simulink model "hyd_hyb_2_opt_cont_1_3"  (Simulink 7.7) (continued)
== system hyd_hyb_2_opt_cont_1_3/controller1/optimized controller/SOC upper 0.5/SOC under 0.6/net_loc_rod_5_6/Layer 2/LW{2,1} == (continued)
      mask Display: e = 0.06;c1 = [120 150 230]/255;c2 = ([120 150 230]+0)/383;c3 = ([120 150 230]+128)/383;patch([0 1 1 0],[0 0 1 1],c1);patch([0 1 1 1-e 1-e 0+e],[0 0 1 1-e 0+e 0+e],c2);patch([0 0 1 1-e 0+e 0+e],[0 1 1 1-e 1-e 0+e],c3);plot([0 1 1 0 0],[0 0 1 1 0]);disp('weights');
  "IW{2,1}(39,:)'" : Constant  Value=[0.051793127253268227;0.0867335350873501;-0.17123112941142279;0.28658186860972723;0.18926556937982511;-0.043935057649215618;0.13359057229240645;-0.047621375948154999;0.18793041389962434;-0.051416531487794681;-0.08856818089903655;-0.38589303828667615;-0.23467469360752585;0.32977369274502261;0.3564742972176565;0.32991702813045037;-0.31138510781671941;0.37081086613078584;0.28676153025468104;-0.30869382703943476;-0.0026450712695630205;-0.26638730282895701;0.38913376997251758;-0.14028202296315356;0.18601520036207678;-0.036540714653514224;0.14064789843506978;-0.14338190033992143;-0.34269091696785714;-0.17620658465693925;0.35632987798502669;-0.22443116239248825;0.32819725097151675;0.27936329078335781;0.081759180577006305;-0.30885710550039658;-0.45341460446711601;-0.078017544448491322;0.45253702671142459;-0.10546329101274068]
      mask Display: e = 0.06;c1 = [120 150 230]/255;c2 = ([120 150 230]+0)/383;c3 = ([120 150 230]+128)/383;patch([0 1 1 0],[0 0 1 1],c1);patch([0 1 1 1-e 1-e 0+e],[0 0 1 1-e 0+e 0+e],c2);patch([0 0 1 1-e 0+e 0+e],[0 1 1 1-e 1-e 0+e],c3);plot([0 1 1 0 0],[0 0 1 1 0]);disp('weights');
  "IW{2,1}(4,:)'" : Constant  Value=[0.26166945625251098;-0.015391374965026268;0.38843456456057973;0.20368367666233722;0.031082817938094705;-0.31984888765571673;-0.021842719752766124;0.23254936809729812;0.3733846428382005;0.17450120067696237;0.25876863857223381;-0.25021254779034552;0.30107195992017444;0.27216368076662156;0.39455419320493268;0.15055949351372644;0.18494408604779197;0.12296440911649757;0.21985512687003991;-0.20846579576787017;-0.07077424242262248;0.28168834350833466;-0.078298909476144443;-0.17164861985544724;-0.25281257372316035;0.32166865434067449;-0.18107836433143712;0.35772334437983455;0.015454886190986684;-0.3660073998703538;-0.099729487379174225;-0.34136163196315983;0.017100924014964922;0.16427923724834531;-0.38652640556843537;-0.06654457955317708;-0.36545535617732722;0.27114312057944767;-0.13280072547520147;-0.23062466165328896]
      mask Display: e = 0.06;c1 = [120 150 230]/255;c2 = ([120 150 230]+0)/383;c3 = ([120 150 230]+128)/383;patch([0 1 1 0],[0 0 1 1],c1);patch([0 1 1 1-e 1-e 0+e],[0 0 1 1-e 0+e 0+e],c2);patch([0 0 1 1-e 0+e 0+e],[0 1 1 1-e 1-e 0+e],c3);plot([0 1 1 0 0],[0 0 1 1 0]);disp('weights');
  "IW{2,1}(40,:)'" : Constant  Value=[0.14480187979545261;-0.41941714327186774;-0.35780211276912016;0.024512038372641577;0.30273435786497532;0.13878043538401386;-0.35159212406694662;-0.25398964794072676;-0.28947420623540304;0.27611795673366452;0.093365299036340638;-0.34334526523495601;0.11648410858136214;-0.1974803030154792;0.056043393075692234;-0.34591718131775501;-0.053546612480675188;0.13915966292225446;0.091872205621835665;-0.0091979654261735364;0.15727455891667225;-0.12268560560427545;-0.20861387387062075;0.28515458061013055;-0.34882815119266358;-0.27003732791152785;0.16164177881052225;0.3239620209002575;-0.23891306562469483;0.15431461217505035;0.33578879978244108;-0.26282892631903626;-0.14778316190202923;0.25256793299625435;-0.070585017727189905;-0.3006923103369778;0.24926829400645109;-0.28719001435833974;-0.12558106315396503;0.4321551170519316]
      mask Display: e = 0.06;c1 = [120 150 230]/255;c2 = ([120 150 230]+0)/383;c3 = ([120 150 230]+128)/383;patch([0 1 1 0],[0 0 1 1],c1);patch([0 1 1 1-e 1-e 0+e],[0 0 1 1-e 0+e 0+e],c2);patch([0 0 1 1-e 0+e 0+e],[0 1 1 1-e 1-e 0+e],c3);plot([0 1 1 0 0],[0 0 1 1 0]);disp('weights');
  "IW{2,1}(41,:)'" : Constant  Value=[-0.32759329641781437;0.24537346203803526;-0.0073491027998336591;0.33835466259436292;0.38067357973857574;-0.14465332964355013;0.19849777553098036;0.042958320966494948;-0.10266019858384033;0.17923280185782203;-0.31973267865946042;-0.39071311864079139;0.045577009861267302;0.39891359788374364;0.19114855963353772;0.18246140083211351;-0.061183244971679762;0.30449648647831018;-0.35902316912602578;-0.31418753242354891;-0.09119721466477837;-0.38729723428796331;-0.11000683388121742;-0.26631129317967878;0.29364103075620229;-0.27496286251298352;-0.048713715870195032;0.094191504555056982;-0.12880845984230224;0.26644173004504396;-0.32953070595739181;0.36656823302284103;-0.12231857491616857;0.066135946501131984;0.25253316837166534;-0.039780405959084469;0.16837903543925417;0.36874169374716059;0.23141980162813247;0.28029026990552897]
      mask Display: e = 0.06;c1 = [120 150 230]/255;c2 = ([120 150 230]+0)/383;c3 = ([120 150 230]+128)/383;patch([0 1 1 0],[0 0 1 1],c1);patch([0 1 1 1-e 1-e 0+e],[0 0 1 1-e 0+e 0+e],c2);patch([0 0 1 1-e 0+e 0+e],[0 1 1 1-e 1-e 0+e],c3);plot([0 1 1 0 0],[0 0 1 1 0]);disp('weights');
  "IW{2,1}(42,:)'" : Constant  Value=[-0.3087562699064354;0.01479828615981137;-0.37792834474962866;0.13677852248658373;-0.32855270204208953;-0.32325921669460389;-0.31798347467554128;0.21839109787669023;-0.31811310472283344;0.07651339820084288;0.19396724780678704;-0.38115232717214287;-0.34556257129549761;0.14007264668724193;0.15716775679286749;-0.37093964357763382;0.28224012417403421;0.14861217562703413;0.22647511515637106;-0.41652314932668055;-0.22201044225525374;-0.35206618803788309;0.085956231256808943;-0.21243863401660087;0.22773383468695901;0.22989319530872215;-0.15369937236401163;0.12308425406712353;-0.32528254046923644;-0.28972870186678595;0.054764188791439052;-0.070186872891953037;0.16638241420368713;0.34228561540854224;0.0048449502598812283;0.17060304529836359;0.21339471190757667;-0.1171274437703435;-0.35326983358716058;0.10782626260251062]
      mask Display: e = 0.06;c1 = [120 150 230]/255;c2 = ([120 150 230]+0)/383;c3 = ([120 150 230]+128)/383;patch([0 1 1 0],[0 0 1 1],c1);patch([0 1 1 1-e 1-e 0+e],[0 0 1 1-e 0+e 0+e],c2);patch([0 0 1 1-e 0+e 0+e],[0 1 1 1-e 1-e 0+e],c3);plot([0 1 1 0 0],[0 0 1 1 0]);disp('weights');
  "IW{2,1}(43,:)'" : Constant  Value=[-0.27671403381418425;0.39497644529486614;0.19884039529498637;-0.024207540201849165;0.3368193130313426;-0.047785363384455118;-0.053348280601332544;0.19191831154073752;-0.28904321521883608;-0.18790579654020279;-0.29625544077472371;-0.24353234951426797;0.099944071485222427;-0.23679764618180843;0.10147430907766201;-0.22027818586511319;0.13584341912346798;-0.25231661471105238;0.25091050704522933;-0.010072938457797123;0.3291327918487657;-0.23398288711799142;-0.36794194806688096;0.010433704228146969;0.1001893720398319;-0.025734088154408617;-0.013144305920785056;-0.13425225101814162;0.29273211599546078;0.35289104932516746;0.32854602239118469;0.3692239932887314;0.3393458848165119;0.10909001070815789;-0.41059103135578612;-0.23851373264167422;0.25116970359433449;-0.21895921531569695;-0.30035182657892689;0.28160258355361861]
      mask Display: e = 0.06;c1 = [120 150 230]/255;c2 = ([120 150 230]+0)/383;c3 = ([120 150 230]+128)/383;patch([0 1 1 0],[0 0 1 1],c1);patch([0 1 1 1-e 1-e 0+e],[0 0 1 1-e 0+e 0+e],c2);patch([0 0 1 1-e 0+e 0+e],[0 1 1 1-e 1-e 0+e],c3);plot([0 1 1 0 0],[0 0 1 1 0]);disp('weights');
  "IW{2,1}(44,:)'" : Constant  Value=[0.065227653002081581;0.37567576744042125;0.20103320961503496;-0.11232882676002968;0.22422593395505538;-0.25013990568364203;0.3976615741642005;0.18900272741947399;-0.21543208604527805;-0.28026511530584153;0.23215890846487516;0.1381062311135694;0.35403485176579924;0.028443255421127717;0.20080551666925714;0.38630286398688396;-0.11558036534184107;0.39335657481233294;0.096464815943382243;-0.070012555922095834;0.18874144124451095;0.23482754072367579;-0.13860133741739722;0.38263286223379006;0.094141169405718206;0.34729770353663736;0.33682673033033583;-0.29747893504632267;-0.34466577024137113;0.010764802663778055;0.17222163154614753;0.20601103947969931;0.14521477526533227;0.11364023619482251;-0.13893097635497781;-0.25249547970694969;0.38702438248298654;0.28124833771607732;0.2124379891606395;-0.18291814755523148]
      mask Display: e = 0.06;c1 = [120 150 230]/255;c2 = ([120 150 230]+0)/383;c3 = ([120 150 230]+128)/383;patch([0 1 1 0],[0 0 1 1],c1);patch([0 1 1 1-e 1-e 0+e],[0 0 1 1-e 0+e 0+e],c2);patch([0 0 1 1-e 0+e 0+e],[0 1 1 1-e 1-e 0+e],c3);plot([0 1 1 0 0],[0 0 1 1 0]);disp('weights');
  "IW{2,1}(45,:)'" : Constant  Value=[-0.081464031813616902;0.238240758316832;0.0042004367062321701;0.18683306169738675;0.22263586522550124;0.29398394728067018;0.28752148452180298;0.26764571612217769;-0.23729322869172653;-0.41556721490699555;0.32126348780488395;0.27007773946099162;-0.023783747835480228;0.29204046065570788;0.26844587062248099;0.27644499197004113;-0.13109666364143963;-0.23911534642184273;-0.39783838378068603;-0.2170966916107597;0.13375792636947173;0.16375513787908644;-0.014400969191436644;0.39863553541260921;0.43155932817639975;-0.26665508454510833;-0.39251517147691362;0.14086978576014386;-0.28151832150872846;0.17177495014893215;-0.032674953675965658;-0.15710461227402442;-0.11630343444076759;-0.032597086898104741;0.26770855307164854;0.1282672531941387;-0.17989830173013766;0.49053183100815639;0.12475772560683679;-0.26983163749503158]
      mask Display: e = 0.06;c1 = [120 150 230]/255;c2 = ([120 150 230]+0)/383;c3 = ([120 150 230]+128)/383;patch([0 1 1 0],[0 0 1 1],c1);patch([0 1 1 1-e 1-e 0+e],[0 0 1 1-e 0+e 0+e],c2);patch([0 0 1 1-e 0+e 0+e],[0 1 1 1-e 1-e 0+e],c3);plot([0 1 1 0 0],[0 0 1 1 0]);disp('weights');
  "IW{2,1}(46,:)'" : Constant  Value=[-0.3131015924298437;0.28708141016942584;-0.017940407307627852;0.12302802936240229;0.015801980627966072;-0.25749936710593385;-0.0017638941688190601;-0.048466674773344154;0.12367087849598908;-0.28411625991077677;0.41300423953876486;0.19804327765225599;-0.0060259105922243891;0.11288894515416228;-0.27024714482357415;0.40065325810964381;-0.18774569556601342;-0.34864070171139888;-0.11793485572002647;-0.34886355389266188;0.041940135584584037;-0.2986546419404244;-0.032786726596024093;0.33284337085533011;-0.43564516977184942;0.19356169082536467;-0.053462716786198641;0.2689344351354116;0.25158389968106465;-0.12519570265187002;0.24248964573726503;0.34709656011161522;-0.36725357003256975;-0.22030911861624797;0.19865683130496931;0.033097178620337037;-0.38746058008191347;-0.03520749260065742;0.28124866971127938;-0.12293306442183935]
      mask Display: e = 0.06;c1 = [120 150 230]/255;c2 = ([120 150 230]+0)/383;c3 = ([120 150 230]+128)/383;patch([0 1 1 0],[0 0 1 1],c1);patch([0 1 1 1-e 1-e 0+e],[0 0 1 1-e 0+e 0+e],c2);patch([0 0 1 1-e 0+e 0+e],[0 1 1 1-e 1-e 0+e],c3);plot([0 1 1 0 0],[0 0 1 1 0]);disp('weights');
  "IW{2,1}(47,:)'" : Constant  Value=[-0.28084672853866371;0.029521095852503572;0.098594644171722048;0.34540163619638953;-0.22640949693072179;0.13310969295224881;0.11174150600891639;-0.2479231339953944;-0.32978391677739266;0.24041616470301058;-0.20251081540029015;0.038090352038501168;-0.25684787491728123;0.24060913613006474;0.40993175496267253;0.45032100795897795;-0.12640075179132948;-0.083741955962267134;-0.13863430319698547;-0.34441848480356158;-0.41165610188065399;-0.26833870881883193;0.19719285230884151;-0.21292078103941722;0.27736549258419557;-0.22561486601889616;-0.35397775628179223;0.003205401375338882;-0.30596818862743269;0.067211218106433213;-0.27497640230046944;0.0039137518864756264;-0.24483615244421036;0.47319103764171394;-0.0068306541671852329;0.21029192332308363;-0.37492174428040442;-0.021206360167852861;0.26184641848369461;-0.12139101255231555]
      mask Display: e = 0.06;c1 = [120 150 230]/255;c2 = ([120 150 230]+0)/383;c3 = ([120 150 230]+128)/383;patch([0 1 1 0],[0 0 1 1],c1);patch([0 1 1 1-e 1-e 0+e],[0 0 1 1-e 0+e 0+e],c2);patch([0 0 1 1-e 0+e 0+e],[0 1 1 1-e 1-e 0+e],c3);plot([0 1 1 0 0],[0 0 1 1 0]);disp('weights');
  "IW{2,1}(48,:)'" : Constant  Value=[-0.2643355616865401;0.34425478114156233;-0.16244865797822977;0.178870552639351;0.15919268671323944;-0.12878378785634878;-0.011951761272175;0.34005816541828277;-0.31406200901536557;-0.36470646467168305;0.37879476345470459;0.34297802880043166;0.14710579412967234;-0.34580216476294418;-0.20861202093781545;0.11893405614546605;0.04261443931693143;-0.13459346405537501;0.062439473613574228;0.22576278634134267;-0.072723684122369819;0.024705465938471109;-0.22770058818618302;0.25354789746539008;0.13772958556774628;0.3892125159691745;0.31032738665266585;-0.073210635317150538;-0.28253162154338313;0.34918467511879187;-0.16869301916580767;-0.28798050605717312;0.37158583474338197;-0.19078079185200386;-0.34518181474522325;-0.09500862056339987;-0.084125022847577821;0.31054245830317218;-0.24142393580183538;-0.24517505407646173]
      mask Display: e = 0.06;c1 = [120 150 230]/255;c2 = ([120 150 230]+0)/383;c3 = ([120 150 230]+128)/383;patch([0 1 1 0],[0 0 1 1],c1);patch([0 1 1 1-e 1-e 0+e],[0 0 1 1-e 0+e 0+e],c2);patch([0 0 1 1-e 0+e 0+e],[0 1 1 1-e 1-e 0+e],c3);plot([0 1 1 0 0],[0 0 1 1 0]);disp('weights');
  "IW{2,1}(49,:)'" : Constant  Value=[-0.10369218368525379;0.16049968045997356;0.1370999202076566;-0.14068193172798396;-0.32437800383064735;0.12048793745400112;-0.13797528738723377;0.4102663384015976;-0.24631564072981954;0.30390743779640328;0.39272975578494773;0.19969541433908775;-0.093264804300024012;-0.23443820833569404;0.055413302705005239;-0.17859731531426826;0.04653566335901986;-0.14671876821235266;-0.24221939829727179;0.14910638093631309;-0.33183066118989824;0.082210028961534246;0.27882495191162654;-0.21029055917758854;0.34852904568376464;-0.20302484038710267;0.35764846378407955;-0.23996418745827103;0.20732496543276474;0.21107884939281563;0.20400430449168336;-0.080346502359988109;-0.054689476995769093;-0.23242548780761571;0.26758456994354907;-0.42923725932552476;-0.40662572287826104;0.273036853482237;-0.2722266549908321;0.36658922899384533]
      mask Display: e = 0.06;c1 = [120 150 230]/255;c2 = ([120 150 230]+0)/383;c3 = ([120 150 230]+128)/383;patch([0 1 1 0],[0 0 1 1],c1);patch([0 1 1 1-e 1-e 0+e],[0 0 1 1-e 0+e 0+e],c2);patch([0 0 1 1-e 0+e 0+e],[0 1 1 1-e 1-e 0+e],c3);plot([0 1 1 0 0],[0 0 1 1 0]);disp('weights');
  "IW{2,1}(5,:)'" : Constant  Value=[0.16055742864868752;0.36647416856062442;0.26446171161236554;0.045070009581576156;0.15199860658008635;0.44335938921561779;0.26738569053125205;-0.12507279109476832;0.33777030918327716;0.24408779449840684;-0.32557199370441714;-0.028932838115301413;0.34436046249352126;-0.12927259289299986;0.35628383106581446;-0.024878291249822954;-0.14238023709580463;0.083958595830212493;-0.089800245230982387;0.22469375917611878;-0.17502194675290383;-0.35678667859132274;0.40091962586710916;-0.098554991294222655;-0.31773333728495701;0.2562016293755075;-0.22645681774596871;-0.30415772206997094;-0.099050001842588581;-0.094775146738007424;-0.1757442165413495;0.24821333940970255;-0.1958521430006073;0.18327496318528899;0.12174265153894498;0.23502474015238597;0.19156809126273147;0.29201913670839935;-0.41021772384697747;0.17114587739684597]
      mask Display: e = 0.06;c1 = [120 150 230]/255;c2 = ([120 150 230]+0)/383;c3 = ([120 150 230]+128)/383;patch([0 1 1 0],[0 0 1 1],c1);patch([0 1 1 1-e 1-e 0+e],[0 0 1 1-e 0+e 0+e],c2);patch([0 0 1 1-e 0+e 0+e],[0 1 1 1-e 1-e 0+e],c3);plot([0 1 1 0 0],[0 0 1 1 0]);disp('weights');
  "IW{2,1}(50,:)'" : Constant  Value=[-0.15281389713634788;0.19062419672170808;-0.34764931787619924;0.27514251865922756;-0.19328601621349023;-0.040597910444059422;-0.40753293783037459;0.21786977827475859;0.20925930737481604;-0.28169193279566002;0.17219248733535616;-0.16653941575701306;0.071061364288123319;-0.38187207662334305;0.05341160547795501;-0.030269865651755878;0.37274176929633573;-0.19809485756408182;0.10354903983592244;-0.18677378857905305;-0.28076214066856958;-0.42442416308118375;0.093905929614936642;-0.01647997833014337;0.19725398594495674;0.28573663385018105;-0.32239719892111185;-0.23563869743960991;-0.27987425149980466;-0.31546418426822903;-0.092061435590861981;0.31952885470934816;0.26531139372623153;0.37589704024124276;0.25525597751551365;-0.33819397117131333;0.20735648181858146;0.045550050317796797;-0.22467748206766908;-0.024869856971619048]
      mask Display: e = 0.06;c1 = [120 150 230]/255;c2 = ([120 150 230]+0)/383;c3 = ([120 150 230]+128)/383;patch([0 1 1 0],[0 0 1 1],c1);patch([0 1 1 1-e 1-e 0+e],[0 0 1 1-e 0+e 0+e],c2);patch([0 0 1 1-e 0+e 0+e],[0 1 1 1-e 1-e 0+e],c3);plot([0 1 1 0 0],[0 0 1 1 0]);disp('weights');
  "IW{2,1}(51,:)'" : Constant  Value=[-0.044834129482377708;-0.28596644997621035;-0.36638378579618314;-0.0091342195695461384;-0.047353762506909883;0.20328889975965753;0.3196062127309488;0.12863310425025226;0.22268708878896676;-0.10533287251400732;0.14453075855950201;-0.013761665837647791;-0.2423664827033524;-0.34345544612576595;0.15259539876913683;-0.37720175340548079;0.15663417235559088;-0.39611062678353115;-0.27866218619558741;-0.060955366354394587;-0.34109232890590802;-0.17640795920486993;0.068692453924288271;-0.41138918674168018;-0.37631516864547526;0.26772645372628145;-0.25863977450137327;-0.2194814658292227;0.38857383268480122;0.2810395270458787;-0.099899804068713974;-0.17557502697715141;-0.03784197548576889;-0.14169457715621939;0.22382566406576296;-0.37536334052825243;-0.11570770939274132;0.21199516775718522;-0.072539333431306974;0.41058533232639743]
      mask Display: e = 0.06;c1 = [120 150 230]/255;c2 = ([120 150 230]+0)/383;c3 = ([120 150 230]+128)/383;patch([0 1 1 0],[0 0 1 1],c1);patch([0 1 1 1-e 1-e 0+e],[0 0 1 1-e 0+e 0+e],c2);patch([0 0 1 1-e 0+e 0+e],[0 1 1 1-e 1-e 0+e],c3);plot([0 1 1 0 0],[0 0 1 1 0]);disp('weights');
  "IW{2,1}(52,:)'" : Constant  Value=[0.29610226928355099;-0.016551889878568649;-0.18573678716326819;0.29365682735367643;-0.31675995480847857;-0.015600600275043532;-0.060557818124216879;0.23340598471150198;-0.055530868688486605;-0.45873372283063224;0.24379819836883376;-0.20383677474585624;0.01605275450075808;-0.093957924037893506;0.20120718185948991;0.034062683585666075;-0.22579940162047293;-0.42600810178110154;-0.37794399384082322;-0.12234782496674226;0.28978146267238364;-0.17660860112317084;-0.042872108592497807;-0.094295931752574516;-0.079834831585061578;0.40282897987164401;0.16622519001995781;0.24193390458753442;-0.24712922873773424;0.36189046547473175;0.34920423861945071;0.13106308355432403;0.22539319235041635;0.31902293455093284;0.28367553675032048;0.22145515159650239;0.033533241739290634;0.30736674793031216;0.31973069897255885;0.3995242330755947]
      mask Display: e = 0.06;c1 = [120 150 230]/255;c2 = ([120 150 230]+0)/383;c3 = ([120 150 230]+128)/383;patch([0 1 1 0],[0 0 1 1],c1);patch([0 1 1 1-e 1-e 0+e],[0 0 1 1-e 0+e 0+e],c2);patch([0 0 1 1-e 0+e 0+e],[0 1 1 1-e 1-e 0+e],c3);plot([0 1 1 0 0],[0 0 1 1 0]);disp('weights');
  "IW{2,1}(53,:)'" : Constant  Value=[-0.11453628898764484;0.40004253573854115;-0.10329560945856374;-0.06568419998566831;-0.14006989641667045;0.11414706558182737;0.36250888389079289;-0.026271731333233746;0.28565770583722361;0.29586454091840519;0.11640137077308103;-0.36668867447573794;-0.23936084675278718;-0.41688021547575238;-0.14492637012579851;-0.21536351483916288;0.44445485025045478;-0.19143427328143597;0.1295561139733967;0.33915378242214911;-0.25260513471644541;0.22363113778798399;-0.16875298893285698;0.29108506296618714;0.34560699685480839;0.27092584503998424;-0.23365303962946501;0.28010670409068811;-0.19228248819718435;-0.010467387166723293;0.224998200538372;0.086826364033216474;-0.26960210679307922;-0.24600525766496167;-0.32694370689473184;0.27708909509041207;0.39490336064886467;-0.14298158353938634;0.12440347582115165;0.36602672096102334]
      mask Display: e = 0.06;c1 = [120 150 230]/255;c2 = ([120 150 230]+0)/383;c3 = ([120 150 230]+128)/383;patch([0 1 1 0],[0 0 1 1],c1);patch([0 1 1 1-e 1-e 0+e],[0 0 1 1-e 0+e 0+e],c2);patch([0 0 1 1-e 0+e 0+e],[0 1 1 1-e 1-e 0+e],c3);plot([0 1 1 0 0],[0 0 1 1 0]);disp('weights');
  "IW{2,1}(54,:)'" : Constant  Value=[-0.33217508339050139;-0.13900554547754446;-0.37607459436904628;0.29417499618771731;-0.011008838896999123;0.021037088807147521;0.11494685360538809;0.0095844321318628023;-0.35207481288704001;-0.47161335401532828;0.42605554140589624;0.44103583338038244;-0.15468903316988117;-0.20114737199132252;-0.026692682681089968;0.017953861455726763;0.090842894224184748;0.2037560645287628;0.38461167827661502;-0.104633195591192;-0.17567422227113472;0.059787155927483716;-0.3660568853190852;0.1015274881005553;-0.31665601473051253;-0.30713376022254718;0.091826944381655437;-0.30169329509841297;0.4327225469202331;0.29908695812390462;0.015494907426369855;0.18061055372520179;0.1042586247075392;-0.074092415641255238;0.28060243793417827;0.0071399279844863005;-0.068007582463831956;0.34252453656393284;-0.12492786083026403;0.13221581067883578]
      mask Display: e = 0.06;c1 = [120 150 230]/255;c2 = ([120 150 230]+0)/383;c3 = ([120 150 230]+128)/383;patch([0 1 1 0],[0 0 1 1],c1);patch([0 1 1 1-e 1-e 0+e],[0 0 1 1-e 0+e 0+e],c2);patch([0 0 1 1-e 0+e 0+e],[0 1 1 1-e 1-e 0+e],c3);plot([0 1 1 0 0],[0 0 1 1 0]);disp('weights');
  "IW{2,1}(55,:)'" : Constant  Value=[0.13206316868607926;-0.13095557376853312;-0.25427119284544281;0.16585857909784729;-0.19413563821325741;-0.060397247828158022;-0.20851166201635471;-0.12026430379917294;0.39355926784507878;0.23558551915172071;-0.31280172049654992;0.18047811280237352;0.38885718028549437;0.33192572102844931;0.39672857357165592;0.28451986184763772;0.36451888965701529;0.17893038241470585;0.38102315780041568;0.1737787185394799;-0.039017142135691234;0.011242315456324599;0.066983506148263358;0.17068670579249215;-0.32249070558685455;0.37708381420735976;-0.32540021659205287;0.33333967816623239;-0.03899088566454996;-0.23683832177967296;-0.20803120344492382;-0.12788047104548614;-0.34422044322861517;-0.049597990506248045;0.056994532122713208;-0.13206724470605;-0.078789912300635312;0.32798456273540216;0.18180876531643475;0.32959084862643184]
      mask Display: e = 0.06;c1 = [120 150 230]/255;c2 = ([120 150 230]+0)/383;c3 = ([120 150 230]+128)/383;patch([0 1 1 0],[0 0 1 1],c1);patch([0 1 1 1-e 1-e 0+e],[0 0 1 1-e 0+e 0+e],c2);patch([0 0 1 1-e 0+e 0+e],[0 1 1 1-e 1-e 0+e],c3);plot([0 1 1 0 0],[0 0 1 1 0]);disp('weights');
  "IW{2,1}(56,:)'" : Constant  Value=[0.43449351604480108;0.38128023742120931;-0.23715988580581598;0.0034285443848455152;-0.3051004183029547;-0.010840640382541532;-0.43463272534441871;0.43360379352972267;-0.47401339356254085;-0.22717740700111155;-0.32880141219397341;-0.46746441472073702;-0.094154298119462035;0.1080330781544028;-0.065269896142724826;-0.044342734538179288;-0.29463254778187753;0.30431942490484737;0.17744253731969264;0.19342950120999733;0.28524128235621254;-0.07135889342569135;0.26075386204060325;0.1914434599099781;-0.005588241421249235;-0.27680645487068939;-0.14977796748975727;-0.16475105417707767;-0.21408000379735725;-0.07438968395935186;-0.13674629476099984;-0.036473030969233954;0.01341551002289708;0.048761102260615197;-0.12396321914253287;0.2447598942929545;-0.44486382694406007;0.016716694621365089;-0.19559748776131355;0.22025685868904515]
      mask Display: e = 0.06;c1 = [120 150 230]/255;c2 = ([120 150 230]+0)/383;c3 = ([120 150 230]+128)/383;patch([0 1 1 0],[0 0 1 1],c1);patch([0 1 1 1-e 1-e 0+e],[0 0 1 1-e 0+e 0+e],c2);patch([0 0 1 1-e 0+e 0+e],[0 1 1 1-e 1-e 0+e],c3);plot([0 1 1 0 0],[0 0 1 1 0]);disp('weights');
  "IW{2,1}(57,:)'" : Constant  Value=[-0.0083745728553244608;0.29476685390391549;0.33262077914476029;0.19653322562095363;0.11454207185404543;-0.34322863929539421;-0.35467575257568884;-0.03054196394628924;-0.31679789362858796;-0.39207677450342338;0.33695879919731436;-0.04593405346293996;0.15488457175891668;-0.37684760863890887;0.1746759653597047;-0.26313310839845239;-0.059354532981901319;0.14331580228799276;0.36797040752388466;-0.34510857449381083;-0.14815903465579042;0.033453469056473471;-0.045638582246678251;0.058509696521560982;-0.05021381888045038;-0.0067222434703053295;-0.30128993564822959;-0.32936309309338674;-0.38911690857055375;0.039634825934388114;0.32105537833767361;0.048933868343075462;0.28927717855369434;0.0099448095300426077;0.1102932069666879;-0.32637681778583011;0.35757459269816311;-0.25620996846653871;-0.32405846714956377;-0.085668009180640298]
      mask Display: e = 0.06;c1 = [120 150 230]/255;c2 = ([120 150 230]+0)/383;c3 = ([120 150 230]+128)/383;patch([0 1 1 0],[0 0 1 1],c1);patch([0 1 1 1-e 1-e 0+e],[0 0 1 1-e 0+e 0+e],c2);patch([0 0 1 1-e 0+e 0+e],[0 1 1 1-e 1-e 0+e],c3);plot([0 1 1 0 0],[0 0 1 1 0]);disp('weights');
  "IW{2,1}(58,:)'" : Constant  Value=[-0.29149125785979185;0.08070714033442565;0.18812190120955219;-0.32638238696815947;0.38728763150981282;-0.37981586507889092;0.12978590289611999;0.039839413213652135;0.12020705201532997;-0.21499443701212853;-0.21568302375121201;0.2388640226988322;-0.30151231575673948;0.15814161811198985;-0.21389779803994363;-0.32632225430957623;0.34302701855415901;0.34504545467679826;0.044895005422193074;-0.12456127431860828;0.22485787027609644;-0.30590200526019479;0.31533177007694524;0.25921948097591446;0.26133261740208641;-0.31973508913637372;-0.25466428104923977;-0.17674476733364849;0.20477978987733522;-0.014160069884654149;0.27715588362438609;-0.11520482262955282;-0.39679291730427879;-0.3401412558818635;-0.10844563083706397;-0.10863543881238494;0.22228821929161124;0.084587878752809084;-0.16412792753187838;-0.28639142307178106]
      mask Display: e = 0.06;c1 = [120 150 230]/255;c2 = ([120 150 230]+0)/383;c3 = ([120 150 230]+128)/383;patch([0 1 1 0],[0 0 1 1],c1);patch([0 1 1 1-e 1-e 0+e],[0 0 1 1-e 0+e 0+e],c2);patch([0 0 1 1-e 0+e 0+e],[0 1 1 1-e 1-e 0+e],c3);plot([0 1 1 0 0],[0 0 1 1 0]);disp('weights');
  "IW{2,1}(59,:)'" : Constant  Value=[0.25636457724538558;-0.04064749452283091;-0.0032783363947652745;-0.32820881605643004;0.29550135018036755;0.082109254120601119;0.25634018646348777;-0.45102079566371212;0.33414575457472556;0.13266312345187581;0.34490489495719295;0.36644779337044725;0.013750974679295831;-0.33828290859329607;-0.3905598154070718;-0.07007745382513153;0.085127922496425729;0.089359162004555112;0.1662071850282994;0.19170161137339073;0.012818803846722729;0.006431316033630465;-0.16379869918044762;-0.065888801552330609;-0.40870016063158482;-0.32373690208955758;-0.39482534237541811;-0.16696615293893427;-0.23727199757621775;0.33879704775218639;-0.35751485051084786;0.0014770915992821005;0.12218129385359579;0.10427221192686932;-0.37009207022807816;0.29829437429388539;-0.28677958990240626;-0.10146392730908625;-0.17317631377829892;-0.019812352015267407]
      mask Display: e = 0.06;c1 = [120 150 230]/255;c2 = ([120 150 230]+0)/383;c3 = ([120 150 230]+128)/383;patch([0 1 1 0],[0 0 1 1],c1);patch([0 1 1 1-e 1-e 0+e],[0 0 1 1-e 0+e 0+e],c2);patch([0 0 1 1-e 0+e 0+e],[0 1 1 1-e 1-e 0+e],c3);plot([0 1 1 0 0],[0 0 1 1 0]);disp('weights');
  "IW{2,1}(6,:)'" : Constant  Value=[-0.30955969531719529;-0.10986787442712617;-0.26712501428413177;0.36541133761382433;-0.37076743912507021;-0.30847618385933828;-0.018189648381940888;-0.077448410772225904;0.34656098503243726;0.25381301284008684;0.15956546972031357;0.32999981244395071;-0.36498872624056944;0.29096500009313897;0.17905588115582044;0.30778659813020443;0.067636679121266793;0.029546116665996609;0.1557311981750131;-0.31540397844205859;-0.10743144340970409;-0.38539202689069196;0.036767637542075002;0.29894030770100166;0.06008732746203397;-0.34084731684653208;0.044816122341888462;-0.28290816056823664;-0.23617670531916327;0.40696764036386268;-0.22484896947516056;0.16558883966127932;0.14073760442608821;0.19511862745258216;0.18901276988659418;0.060092308497508184;-0.25547094347016291;0.27116250314831297;-0.13806582680663976;0.036929388514358535]
      mask Display: e = 0.06;c1 = [120 150 230]/255;c2 = ([120 150 230]+0)/383;c3 = ([120 150 230]+128)/383;patch([0 1 1 0],[0 0 1 1],c1);patch([0 1 1 1-e 1-e 0+e],[0 0 1 1-e 0+e 0+e],c2);patch([0 0 1 1-e 0+e 0+e],[0 1 1 1-e 1-e 0+e],c3);plot([0 1 1 0 0],[0 0 1 1 0]);disp('weights');
  "IW{2,1}(60,:)'" : Constant  Value=[-0.10219728922827656;-0.26727183264254656;0.063116849797940633;0.13477773911427879;0.060254065703592923;0.2442530329419044;-0.22422261113437139;-0.17624133107662213;0.37424127439873606;-0.21049410308949676;0.29892696477958852;0.32308017098279673;0.21704915283409237;0.10125818751998972;-0.40117401015295284;-0.21530980711315945;0.33212505105061596;0.27003743548852072;-0.014563905297295454;-0.043428819802400528;0.31941419862810361;-0.30479846644638425;0.061452001461875475;0.31929334683281191;-0.33356094787898188;-0.27740523037167536;-0.14022605703943641;0.16827616224608777;0.32578062066471181;0.026608232911129428;-0.21994363685375756;0.39159423618004324;-0.37979117957367875;0.098682612362646124;-0.37466272499700515;0.37209002458882035;0.17020068457114118;0.21041230683435286;0.29510908416786436;-0.1438470021765825]
      mask Display: e = 0.06;c1 = [120 150 230]/255;c2 = ([120 150 230]+0)/383;c3 = ([120 150 230]+128)/383;patch([0 1 1 0],[0 0 1 1],c1);patch([0 1 1 1-e 1-e 0+e],[0 0 1 1-e 0+e 0+e],c2);patch([0 0 1 1-e 0+e 0+e],[0 1 1 1-e 1-e 0+e],c3);plot([0 1 1 0 0],[0 0 1 1 0]);disp('weights');
  "IW{2,1}(7,:)'" : Constant  Value=[0.13354653953659223;0.3687102365019958;0.28634862125021898;0.37684569823125363;0.22401738867012258;-0.022765324816638257;-0.37674725261436232;-0.25929014529613892;0.20582038860585583;0.15751709049831142;-0.25345829414238114;-0.26190484864752317;0.23199371932912036;0.19223385058274312;-0.084534901096333825;-0.12675685387213104;-0.16009602570550296;-0.32112629772416001;-0.13202760819357082;-0.19691365094924096;-0.37979246584836551;0.40510270357279216;0.30860116703203588;-0.27162714509813368;0.19657628326827717;-0.15909309143245701;-0.32609194315055912;-0.048279870580227029;0.086797250536747167;0.3447393758161828;-0.045600036418953535;0.14544476088068897;0.10774615448023141;0.32513421600812498;0.23353759451311992;0.37788876770981883;0.13299211865478869;0.20631139028328302;0.12903718678604056;-0.27507249345165086]
      mask Display: e = 0.06;c1 = [120 150 230]/255;c2 = ([120 150 230]+0)/383;c3 = ([120 150 230]+128)/383;patch([0 1 1 0],[0 0 1 1],c1);patch([0 1 1 1-e 1-e 0+e],[0 0 1 1-e 0+e 0+e],c2);patch([0 0 1 1-e 0+e 0+e],[0 1 1 1-e 1-e 0+e],c3);plot([0 1 1 0 0],[0 0 1 1 0]);disp('weights');
  "IW{2,1}(8,:)'" : Constant  Value=[0.28746083212739132;0.44766539390378596;0.41629772437026624;0.18453488579142421;0.28883808518962917;0.17297342704773938;0.041647382702848547;-0.16984459105530275;0.21308075830960901;0.2665141047141249;-0.33214626248781121;0.23874313706826863;0.13676062172713566;-0.33947436100650707;-0.1102932718565028;0.0032730066386971808;-0.20144134038583789;0.014449116872080416;0.23762957562646495;-0.35772287898530952;-0.37818368887515041;0.15800214658819686;-0.12626154682898322;0.17291279448175234;-0.32147595246229332;0.23545411246497983;0.065031361903296628;-0.1286026352211605;0.15838575220360729;0.22152273860313679;0.3586901246398071;-0.17680198739051894;-0.18767348272978962;0.024173063048223736;-0.02437453649748941;0.21220206517456908;0.24549673404579961;0.29548756457549885;-0.27836934225209808;-0.41236633110503151]
      mask Display: e = 0.06;c1 = [120 150 230]/255;c2 = ([120 150 230]+0)/383;c3 = ([120 150 230]+128)/383;patch([0 1 1 0],[0 0 1 1],c1);patch([0 1 1 1-e 1-e 0+e],[0 0 1 1-e 0+e 0+e],c2);patch([0 0 1 1-e 0+e 0+e],[0 1 1 1-e 1-e 0+e],c3);plot([0 1 1 0 0],[0 0 1 1 0]);disp('weights');
  "IW{2,1}(9,:)'" : Constant  Value=[0.056421987938368445;0.43051209121193801;-0.3177636045668269;0.015195481542260436;0.002009567801928363;-0.12643216616039726;0.11040480047567242;-0.29921226080484603;0.13166219139945651;-0.42419942446073738;-0.26276328874254928;0.01241966952745441;0.038295682332895167;-0.27949392557675939;-0.19140643956849898;-0.2423638287239458;-0.4384301356288024;0.075895123224735059;-0.25948247928180085;-0.12059537446297611;-0.39135991625943956;0.24631872462321625;-0.094520233292546371;0.25762259679894017;0.19321668787834798;0.32673708796781814;-0.3017716102427998;0.058908786729054592;-0.39175769668372978;0.36788628681445579;0.068188774178362679;-0.08094218960639224;0.13526100636190302;-0.23359756637694429;-0.22104449204308332;0.38049963094761075;-0.26220535878296353;-0.074783723679575864;-0.30623974935948578;-0.29893377007521543]
      mask Display: e = 0.06;c1 = [120 150 230]/255;c2 = ([120 150 230]+0)/383;c3 = ([120 150 230]+128)/383;patch([0 1 1 0],[0 0 1 1],c1);patch([0 1 1 1-e 1-e 0+e],[0 0 1 1-e 0+e 0+e],c2);patch([0 0 1 1-e 0+e 0+e],[0 1 1 1-e 1-e 0+e],c3);plot([0 1 1 0 0],[0 0 1 1 0]);disp('weights');
  Mux : Mux  Inputs=60
      mask Display: e = 0.06;c1 = [120 150 230]/255;c2 = ([120 150 230]+0)/383;c3 = ([120 150 230]+128)/383;patch([0 1 1 0],[0 0 1 1],c1);patch([0 1 1 1-e 1-e 0+e],[0 0 1 1-e 0+e 0+e],c2);patch([0 0 1 1-e 0+e 0+e],[0 1 1 1-e 1-e 0+e],c3);plot([0 1 1 0 0],[0 0 1 1 0]);disp('Mux');
  dotprod{1..60} (60 alike) : neural/Weight Functions/dotprod  SystemSampleTime=-1
  "lz{2,1}" : Outport
      mask Display: e = 0.06;c1 = [120 230 180]/255;c2 = ([120 230 180]+0)/(256+128);c3 = ([120 230 180]+128)/(256+128);patch([0 1 1 0],[0 0 1 1],c1);patch([0 1 1 1-e 1-e 0+e],[0 0 1 1-e 0+e 0+e],c2);patch([0 0 1 1-e 0+e 0+e],[0 1 1 1-e 1-e 0+e],c3);plot([0 1 1 0 0],[0 0 1 1 0]);
  wires:
  "IW{2,1}(1,:)'"/1 -> dotprod1/1
  "ad{2,1}"/1 -> dotprod1/2, dotprod2/2, dotprod3/2, dotprod4/2, dotprod5/2, dotprod6/2, dotprod7/2, dotprod8/2, dotprod9/2, dotprod10/2, dotprod11/2, dotprod12/2, dotprod13/2, dotprod14/2, dotprod15/2, dotprod16/2, dotprod17/2, dotprod18/2, dotprod19/2, dotprod20/2, dotprod21/2, dotprod22/2, dotprod23/2, dotprod24/2, dotprod25/2, dotprod26/2, dotprod27/2, dotprod28/2, dotprod29/2, dotprod30/2, dotprod31/2, dotprod32/2, dotprod33/2, dotprod34/2, dotprod35/2, dotprod36/2, dotprod37/2, dotprod38/2, dotprod39/2, dotprod40/2, dotprod41/2, dotprod42/2, dotprod43/2, dotprod44/2, dotprod45/2, dotprod46/2, dotprod47/2, dotprod48/2, dotprod49/2, dotprod50/2, dotprod51/2, dotprod52/2, dotprod53/2, dotprod54/2, dotprod55/2, dotprod56/2, dotprod57/2, dotprod58/2, dotprod59/2, dotprod60/2
  dotprod1/1 -> Mux/1
  "IW{2,1}(2,:)'"/1 -> dotprod2/1
  dotprod2/1 -> Mux/2
  "IW{2,1}(3,:)'"/1 -> dotprod3/1
  dotprod3/1 -> Mux/3
  "IW{2,1}(4,:)'"/1 -> dotprod4/1
  dotprod4/1 -> Mux/4
  "IW{2,1}(5,:)'"/1 -> dotprod5/1
  dotprod5/1 -> Mux/5
  "IW{2,1}(6,:)'"/1 -> dotprod6/1
  dotprod6/1 -> Mux/6
  "IW{2,1}(7,:)'"/1 -> dotprod7/1
  dotprod7/1 -> Mux/7
  "IW{2,1}(8,:)'"/1 -> dotprod8/1
  dotprod8/1 -> Mux/8
  "IW{2,1}(9,:)'"/1 -> dotprod9/1
  dotprod9/1 -> Mux/9
  "IW{2,1}(10,:)'"/1 -> dotprod10/1
  dotprod10/1 -> Mux/10
  "IW{2,1}(11,:)'"/1 -> dotprod11/1
  dotprod11/1 -> Mux/11
  "IW{2,1}(12,:)'"/1 -> dotprod12/1
  dotprod12/1 -> Mux/12
  "IW{2,1}(13,:)'"/1 -> dotprod13/1
  dotprod13/1 -> Mux/13
  "IW{2,1}(14,:)'"/1 -> dotprod14/1
  dotprod14/1 -> Mux/14
  "IW{2,1}(15,:)'"/1 -> dotprod15/1
  dotprod15/1 -> Mux/15
  "IW{2,1}(16,:)'"/1 -> dotprod16/1
  dotprod16/1 -> Mux/16
  "IW{2,1}(17,:)'"/1 -> dotprod17/1
  dotprod17/1 -> Mux/17
  "IW{2,1}(18,:)'"/1 -> dotprod18/1
  dotprod18/1 -> Mux/18
  "IW{2,1}(19,:)'"/1 -> dotprod19/1
  dotprod19/1 -> Mux/19
  "IW{2,1}(20,:)'"/1 -> dotprod20/1
  dotprod20/1 -> Mux/20
  "IW{2,1}(21,:)'"/1 -> dotprod21/1
  dotprod21/1 -> Mux/21
  "IW{2,1}(22,:)'"/1 -> dotprod22/1
  dotprod22/1 -> Mux/22
  "IW{2,1}(23,:)'"/1 -> dotprod23/1
  dotprod23/1 -> Mux/23
  "IW{2,1}(24,:)'"/1 -> dotprod24/1
  dotprod24/1 -> Mux/24
  "IW{2,1}(25,:)'"/1 -> dotprod25/1
  dotprod25/1 -> Mux/25
  "IW{2,1}(26,:)'"/1 -> dotprod26/1
  dotprod26/1 -> Mux/26
  "IW{2,1}(27,:)'"/1 -> dotprod27/1
  dotprod27/1 -> Mux/27
  "IW{2,1}(28,:)'"/1 -> dotprod28/1
  dotprod28/1 -> Mux/28
  "IW{2,1}(29,:)'"/1 -> dotprod29/1
  dotprod29/1 -> Mux/29
  "IW{2,1}(30,:)'"/1 -> dotprod30/1
  dotprod30/1 -> Mux/30
  "IW{2,1}(31,:)'"/1 -> dotprod31/1
  dotprod31/1 -> Mux/31
  "IW{2,1}(32,:)'"/1 -> dotprod32/1
  dotprod32/1 -> Mux/32
  "IW{2,1}(33,:)'"/1 -> dotprod33/1
  dotprod33/1 -> Mux/33
  "IW{2,1}(34,:)'"/1 -> dotprod34/1
  dotprod34/1 -> Mux/34
  "IW{2,1}(35,:)'"/1 -> dotprod35/1
  dotprod35/1 -> Mux/35
  "IW{2,1}(36,:)'"/1 -> dotprod36/1
  dotprod36/1 -> Mux/36
  "IW{2,1}(37,:)'"/1 -> dotprod37/1
  dotprod37/1 -> Mux/37
  "IW{2,1}(38,:)'"/1 -> dotprod38/1
  dotprod38/1 -> Mux/38
  "IW{2,1}(39,:)'"/1 -> dotprod39/1
  dotprod39/1 -> Mux/39
  "IW{2,1}(40,:)'"/1 -> dotprod40/1
  dotprod40/1 -> Mux/40
  "IW{2,1}(41,:)'"/1 -> dotprod41/1
  dotprod41/1 -> Mux/41
  "IW{2,1}(42,:)'"/1 -> dotprod42/1
  dotprod42/1 -> Mux/42
  "IW{2,1}(43,:)'"/1 -> dotprod43/1
  dotprod43/1 -> Mux/43
  "IW{2,1}(44,:)'"/1 -> dotprod44/1
  dotprod44/1 -> Mux/44
  "IW{2,1}(45,:)'"/1 -> dotprod45/1
  dotprod45/1 -> Mux/45
  "IW{2,1}(46,:)'"/1 -> dotprod46/1
  dotprod46/1 -> Mux/46
  "IW{2,1}(47,:)'"/1 -> dotprod47/1
  dotprod47/1 -> Mux/47
  "IW{2,1}(48,:)'"/1 -> dotprod48/1
  dotprod48/1 -> Mux/48
  "IW{2,1}(49,:)'"/1 -> dotprod49/1
  dotprod49/1 -> Mux/49
  "IW{2,1}(50,:)'"/1 -> dotprod50/1
  dotprod50/1 -> Mux/50
  "IW{2,1}(51,:)'"/1 -> dotprod51/1
  dotprod51/1 -> Mux/51
  "IW{2,1}(52,:)'"/1 -> dotprod52/1
  dotprod52/1 -> Mux/52
  "IW{2,1}(53,:)'"/1 -> dotprod53/1
  dotprod53/1 -> Mux/53
  "IW{2,1}(54,:)'"/1 -> dotprod54/1
  dotprod54/1 -> Mux/54
  "IW{2,1}(55,:)'"/1 -> dotprod55/1
  dotprod55/1 -> Mux/55
  "IW{2,1}(56,:)'"/1 -> dotprod56/1
  dotprod56/1 -> Mux/56
  "IW{2,1}(57,:)'"/1 -> dotprod57/1
  dotprod57/1 -> Mux/57
  "IW{2,1}(58,:)'"/1 -> dotprod58/1
  dotprod58/1 -> Mux/58
  "IW{2,1}(59,:)'"/1 -> dotprod59/1
  dotprod59/1 -> Mux/59
  "IW{2,1}(60,:)'"/1 -> dotprod60/1
  dotprod60/1 -> Mux/60
  Mux/1 -> "lz{2,1}"/1
